simulink model "ClimaEgyMgr"  (R2022b)
settings: Solver=FixedStepDiscrete  StartTime=0.0  StopTime=10.0  FixedStep=0.2  RelTol=1e-3
(part 1 of 2)
settings Model: Creator=blue.ge  LastModifiedBy=blue.ge
settings DataIOCC: SaveFormat=StructureWithTime
settings OptimizationCC: DefaultParameterBehavior=Inlined  EnhancedBackFolding=on  PassReuseOutputArgsAs=Individual arguments  ZeroExternalMemoryAtStartup=off  ZeroInternalMemoryAtStartup=off  OptimizeBlockOrder=speed  DifferentSizesBufferReuse=on  OptimizationCustomize=off  EfficientTunableParamExpr=on
settings DebuggingCC: ParameterTunabilityLossMsg=error
settings ModelReferenceCC: ModelReferenceNumInstancesAllowed=Single
settings SFSimCC: MATLABDynamicMemAlloc=off
settings RTWCC: Description=AUTOSAR  SystemTargetFile=autosar.tlc  GenCodeOnly=on  TemplateMakefile=ert_default_tmf
settings CodeAppCC: ForceParamTrailComments=on  IgnoreCustomStorageClasses=off  OperatorAnnotations=on  SimulinkDataObjDesc=on  SFDataObjDesc=on  InsertBlockDesc=on
settings STFCustomTargetCC: TargetLangStandard=C99 (ISO)  MultiwordLength=256  ParMdlRefBuildCompliant=on  CompOptLevelCompliant=on  IncludeMdlTerminateFcn=off  CombineOutputUpdateFcns=on  SuppressErrorStatus=on  ERTCustomFileBanners=on  SupportNonFinite=off  SupportComplex=off  SupportContinuousTime=off  SupportNonInlinedSFcns=off  RemoveResetFunc=on  MATLABClassNameForMDSCustomization=Simulink.SoftwareTarget.ERTCustomization  AutosarCompliant=on  UseToolchainInfoCompliant=on  LookupTableObjectStructAxisOrder=2,1,3,4,...  LUTObjectStructOrderEvenSpacing=Size,Table,Breakpoints  SystemTargetFile=autosar.tlc
settings ERTTargetCC: TargetLangStandard=C99 (ISO)  ConcurrentExecutionCompliant=off  IncludeMdlTerminateFcn=off  SuppressErrorStatus=on  SupportNonFinite=off  SupportComplex=off  ModelStepFunctionPrototypeControlCompliant=off  CPPClassGenCompliant=off  AutosarCompliant=on  LookupTableObjectStructAxisOrder=2,1,3,4,...  LUTObjectStructOrderEvenSpacing=Size,Table,Breakpoints  InlinedParameterPlacement=NonHierarchical  ERTCustomFileTemplate=example_file_process.tlc

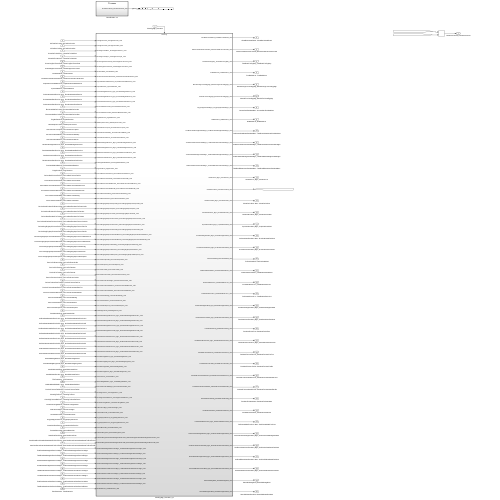
[thumbnail: Simulink's stored preview of the top-level diagram]
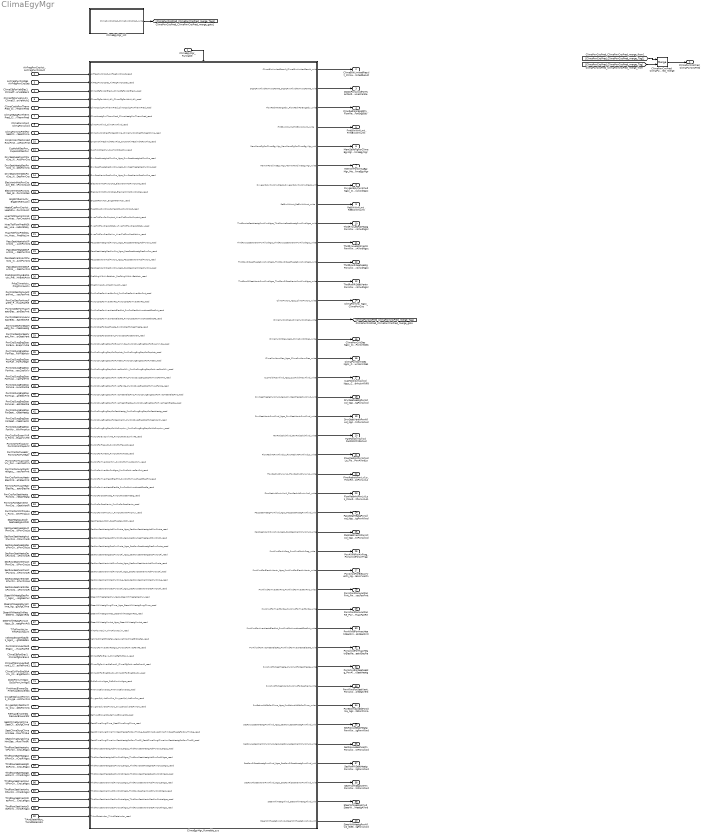
== system ClimaEgyMgr ==
  ClimaEgyMgr_Runnable : Inport  SampleTime=0.2  OutputFunctionCall=on
  AirFragPwrCnsAct_AirFragPwrCnsAct : Inport  Port=2  OutDataTypeStr=Pwr33  PortDimensions=1
  AirFragPwrCnsDes_AirFragPwrCnsDes : Inport  Port=3  OutDataTypeStr=Pwr33  PortDimensions=1
  ClimaCfgForVehElec1_ClimaCfgForVehElec1 : Inport  Port=4  OutDataTypeStr=Boolean  PortDimensions=1
  ClimaCfgForVehHyb1_ClimaCfgForVehHyb1 : Inport  Port=5  OutDataTypeStr=Boolean  PortDimensions=1
  ClimaCoolgPwrThermPred_ClimaCoolgPwrThermPred : Inport  Port=6  OutDataTypeStr=Bus: ClimaCoolgPwrThermPred  PortDimensions=1  BusOutputAsStruct=on
  ClimaHeatgPwrThermPred_ClimaHeatgPwrThermPred : Inport  Port=7  OutDataTypeStr=Bus: ClimaHeatgPwrThermPred  PortDimensions=1  BusOutputAsStruct=on
  ClimaPwrAllwd_ClimaPwrAllwd : Inport  Port=8  OutMin=0  OutMax=2047  OutDataTypeStr=Pwr30  PortDimensions=1
  ClimaPwrCnsPredForSeatClima_ClimaPwrCnsPredForSeatClima : Inport  Port=9  OutDataTypeStr=Bus: ClimaPwrCnsPredRec1  PortDimensions=1  BusOutputAsStruct=on
  CmptmtAirTEstimdAtRowFirst_CmptmtAirTEstimdAtRowFirst : Inport  Port=10  OutDataTypeStr=T30  PortDimensions=1
  CupHoldrDesPwr_CupHoldrDesPwr : Inport  Port=11  OutDataTypeStr=UInt7  PortDimensions=1
  DrvrSeatHeatgActlPwrCns_Ngcc_DrvrSeatHeatgActlPwrCns : Inport  Port=12  OutDataTypeStr=UInt8  PortDimensions=1
  DrvrSeatHeatgDesPwrCns_Ngcc_DrvrSeatHeatgDesPwrCns : Inport  Port=13  OutDataTypeStr=UInt8  PortDimensions=1
  DrvrSeatVentnDesPwrCns_Ngcc_DrvrSeatVentnDesPwrCns : Inport  Port=14  OutDataTypeStr=UInt8  PortDimensions=1
  ElecVentnMotPwrCnsAct_ElecVentnMotPwrCnsAct : Inport  Port=15  OutDataTypeStr=ScrHeatrEgyPwrCns1  PortDimensions=1
  ElecVentnMotPwrCnsDes_ElecVentnMotPwrCnsDes : Inport  Port=16  OutDataTypeStr=ScrHeatrEgyPwrCns1  PortDimensions=1
  EngStrtRemActv_EngStrtRemActv : Inport  Port=17  OutDataTypeStr=Boolean  PortDimensions=1
  HeatdCupPwrCnsAct_HeatdCupPwrCnsAct : Inport  Port=18  OutDataTypeStr=UInt7  PortDimensions=1
  HvacTotFlowForCmptmt_HvacTotFlowForCmptmt : Inport  Port=19  OutDataTypeStr=AirFlow30  PortDimensions=1
  HvacTotFlowPredAtStaby_HvacTotFlowPredAtStaby : Inport  Port=20  OutDataTypeStr=AirFlow30  PortDimensions=1
  HvacTotFlowPredNoLim_HvacTotFlowPredNoLim : Inport  Port=21  OutDataTypeStr=AirFlow30  PortDimensions=1
  PassSeatHeatgActlPwrCns_Ngcc_PassSeatHeatgActlPwrCns : Inport  Port=22  OutDataTypeStr=UInt8  PortDimensions=1
  PassSeatHeatgDesPwrCns_Ngcc_PassSeatHeatgDesPwrCns : Inport  Port=23  OutDataTypeStr=UInt8  PortDimensions=1
  PassSeatVentnActlPwrCns_Ngcc_PassSeatVentnActlPwrCns : Inport  Port=24  OutDataTypeStr=UInt8  PortDimensions=1
  PassSeatVentnDesPwrCns_Ngcc_PassSeatVentnDesPwrCns : Inport  Port=25  OutDataTypeStr=UInt8  PortDimensions=1
  PreCdngWithHvBattActv_PreCdngWithHvBattActv : Inport  Port=26  OutDataTypeStr=Boolean  PortDimensions=1
  PrkgClimaActv_PrkgClimaActv : Inport  Port=27  OutDataTypeStr=Enum: PrkgClimaActv  PortDimensions=1
  PwrCnsDesForHvacFanFrnt_PwrCnsDesForHvacFanFrnt : Inport  Port=28  OutDataTypeStr=Pwr33  PortDimensions=1
  PwrCnsDesForHvacFanRe_PwrCnsDesForHvacFanRe : Inport  Port=29  OutDataTypeStr=Pwr33  PortDimensions=1
  PwrCnsDesForHvacHeatrElecFrnt_PwrCnsDesForHvacHeatrElecFrnt : Inport  Port=30  OutDataTypeStr=Pwr33  PortDimensions=1
  PwrCnsDesForHvacHeatrElecRe_PwrCnsDesForHvacHeatrElecRe : Inport  Port=31  OutDataTypeStr=Pwr33  PortDimensions=1
  PwrCnsDesForSeatHeatg_PwrCnsDesForSeatHeatg : Inport  Port=32  OutDataTypeStr=Pwr33  PortDimensions=1
  PwrCnsDesForSeatVent_PwrCnsDesForSeatVent : Inport  Port=33  OutDataTypeStr=Pwr33  PortDimensions=1
  PwrCnsDurgEngStopForEvaprVlvRe_PwrCnsDurgEngStopForEvaprVlvRe : Inport  Port=34  OutDataTypeStr=Pwr33  PortDimensions=1
  PwrCnsDurgEngStopForFlapActr_PwrCnsDurgEngStopForFlapActr : Inport  Port=35  OutDataTypeStr=Pwr33  PortDimensions=1
  PwrCnsDurgEngStopForFuHeatr_PwrCnsDurgEngStopForFuHeatr : Inport  Port=36  OutDataTypeStr=Pwr33  PortDimensions=1
  PwrCnsDurgEngStopForHvacCooltVlv_PwrCnsDurgEngStopForHvacCooltVlv : Inport  Port=37  OutDataTypeStr=Pwr33  PortDimensions=1
  PwrCnsDurgEngStopForHvacFanFrnt_PwrCnsDurgEngStopForHvacFanFrnt : Inport  Port=38  OutDataTypeStr=Pwr33  PortDimensions=1
  PwrCnsDurgEngStopForHvacFanRe_PwrCnsDurgEngStopForHvacFanRe : Inport  Port=39  OutDataTypeStr=Pwr33  PortDimensions=1
  PwrCnsDurgEngStopForHvacHeatrElecFrnt_PwrCnsDurgEngStopForHvacHeatrElecFrnt : Inport  Port=40  OutDataTypeStr=Pwr33  PortDimensions=1
  PwrCnsDurgEngStopForHvacHeatrElecRe_PwrCnsDurgEngStopForHvacHeatrElecRe : Inport  Port=41  OutDataTypeStr=Pwr33  PortDimensions=1
  PwrCnsDurgEngStopForSeatHeatg_PwrCnsDurgEngStopForSeatHeatg : Inport  Port=42  OutDataTypeStr=Pwr33  PortDimensions=1
  PwrCnsDurgEngStopForSeatVentn_PwrCnsDurgEngStopForSeatVentn : Inport  Port=43  OutDataTypeStr=Pwr33  PortDimensions=1
  PwrCnsDurgEngStopForWtrPmpAux_PwrCnsDurgEngStopForWtrPmpAux : Inport  Port=44  OutDataTypeStr=Pwr33  PortDimensions=1
  PwrCnsForEvaprVlvRe_PwrCnsForEvaprVlvRe : Inport  Port=45  OutDataTypeStr=Pwr33  PortDimensions=1
  PwrCnsForFlapActr_PwrCnsForFlapActr : Inport  Port=46  OutDataTypeStr=Pwr33  PortDimensions=1
  PwrCnsForFuHeatr_PwrCnsForFuHeatr : Inport  Port=47  OutDataTypeStr=Pwr33  PortDimensions=1
  PwrCnsForHvacCooltVlv_PwrCnsForHvacCooltVlv : Inport  Port=48  OutDataTypeStr=Pwr33  PortDimensions=1
  PwrCnsForHvacFanFrntNgcc_PwrCnsForHvacFanFrnt : Inport  Port=49  OutDataTypeStr=Pwr33  PortDimensions=1
  PwrCnsForHvacHeatrElecFrnt_PwrCnsForHvacHeatrElecFrnt : Inport  Port=50  OutDataTypeStr=Pwr33  PortDimensions=1
  PwrCnsForHvacHeatrElecRe_PwrCnsForHvacHeatrElecRe : Inport  Port=51  OutDataTypeStr=Pwr33  PortDimensions=1
  PwrCnsForSeatHeatg_PwrCnsForSeatHeatg : Inport  Port=52  OutDataTypeStr=Pwr33  PortDimensions=1
  PwrCnsForSeatVentn_PwrCnsForSeatVentn : Inport  Port=53  OutDataTypeStr=Pwr33  PortDimensions=1
  PwrCnsForWtrPmpAux_PwrCnsForWtrPmpAux : Inport  Port=54  OutDataTypeStr=Pwr33  PortDimensions=1
  SeatHeatgAutCdn_SeatHeatgAutCdn : Inport  Port=55  OutDataTypeStr=Enum: Flg1  PortDimensions=1
  SecRowSeatHeatgActlPwrCnsLe_Ngcc_SecRowSeatHeatgActlPwrCnsLe : Inport  Port=56  OutDataTypeStr=UInt8  PortDimensions=1
  SecRowSeatHeatgActlPwrCnsRi_Ngcc_SecRowSeatHeatgActlPwrCnsRi : Inport  Port=57  OutDataTypeStr=UInt8  PortDimensions=1
  SecRowSeatHeatgDesPwrCnsLe_Ngcc_SecRowSeatHeatgDesPwrCnsLe : Inport  Port=58  OutDataTypeStr=UInt8  PortDimensions=1
  SecRowSeatHeatgDesPwrCnsRi_Ngcc_SecRowSeatHeatgDesPwrCnsRi : Inport  Port=59  OutDataTypeStr=UInt8  PortDimensions=1
  SecRowSeatVentnActlPwrCnsLe_Ngcc_SecRowSeatVentnActlPwrCnsLe : Inport  Port=60  OutDataTypeStr=UInt8  PortDimensions=1
  SecRowSeatVentnActlPwrCnsRi_Ngcc_SecRowSeatVentnActlPwrCnsRi : Inport  Port=61  OutDataTypeStr=UInt8  PortDimensions=1
  SecRowSeatVentnDesPwrCnsLe_Ngcc_SecRowSeatVentnDesPwrCnsLe : Inport  Port=62  OutDataTypeStr=UInt8  PortDimensions=1
  SecRowSeatVentnDesPwrCnsRi_Ngcc_SecRowSeatVentnDesPwrCnsRi : Inport  Port=63  OutDataTypeStr=UInt8  PortDimensions=1
  SteerWhlHeatgDesPwr_Ngcc_SteerWhlHeatgDesPwr : Inport  Port=64  OutDataTypeStr=UInt8  PortDimensions=1
  SteerWhlHeatgDurgClima_Ngcc_SteerWhlHeatgDurgClima : Inport  Port=65  OutDataTypeStr=Boolean  PortDimensions=1
  SteerWhlHeatgOnReq_SteerWhlHeatgOnReq : Inport  Port=66  OutDataTypeStr=Enum: SteerWhlHeatgOnCmdTyp  PortDimensions=1
  SteerWhlHeatgPwrAct_Ngcc_SteerWhlHeatgPwrAct : Inport  Port=67  OutDataTypeStr=UInt8  PortDimensions=1
  TiToFlowNoLim_TiToFlowNoLim : Inport  Port=68  OutDataTypeStr=Ti33  PortDimensions=1
  VehModMngtGlbSafe1_Ngcc_VehModMngtGlbSafe1 : Inport  Port=69  OutDataTypeStr=Bus: VehModMngtGlbSafe1  PortDimensions=1  BusOutputAsStruct=on
  PwrCnsForHvacFanReNgcc_PwrCnsForHvacFanRe : Inport  Port=70  OutDataTypeStr=Pwr33  PortDimensions=1
  ClimaCfgForErev1_ClimaCfgForErev1 : Inport  Port=71  OutDataTypeStr=Boolean  PortDimensions=1
  ClimaCfgForHvacRePrsnt1_ClimaCfgForHvacRePrsnt1 : Inport  Port=72  OutDataTypeStr=Enum: CarCfgForHvacRe  PortDimensions=1
  ClimaCtrlForEngStbActv_ClimaCtrlForEngStbActv : Inport  Port=73  OutDataTypeStr=Boolean  PortDimensions=1
  DcDcPwrLimNgcc_DcDcPwrLimNgcc : Inport  Port=74  OutDataTypeStr=Boolean  PortDimensions=1
  FrntHvacBlowerSts_FrntHvacBlowerSts : Inport  Port=75  OutDataTypeStr=Enum: HvacFanSts  PortDimensions=1
  OxygenSplyActPwrCns_OxygenSplyActPwrCns : Inport  Port=76  OutMin=0  OutMax=2047  OutDataTypeStr=Pwr30  PortDimensions=1
  OxygenSplyDesPwrCns_OxygenSplyDesPwrCns : Inport  Port=77  OutMin=0  OutMax=2047  OutDataTypeStr=Pwr30  PortDimensions=1
  ReHvacBlowerSts_ReHvacBlowerSts : Inport  Port=78  OutDataTypeStr=Enum: HvacFanSts  PortDimensions=1
  SeatClimaDurgClima_SeatClimaDurgClima : Inport  Port=79  OutDataTypeStr=Bus: HmiSeatClima  PortDimensions=1  BusOutputAsStruct=on
  SeatClimaDurgClimaHmiSeatHeatgForRowThrdLe_SeatClimaDurgClimaHmiSeatHeatgForRowThrdLe : Inport  Port=80  OutDataTypeStr=Enum: SeatClimaLvl  PortDimensions=1
  SeatClimaDurgClimaHmiSeatHeatgForRowThrdRi_SeatClimaDurgClimaHmiSeatHeatgForRowThrdRi : Inport  Port=81  OutDataTypeStr=Enum: SeatClimaLvl  PortDimensions=1
  ThrdRowSeatHeatgActlPwrCnsLeNgcc_ThrdRowSeatHeatgActlPwrCnsLeNgcc : Inport  Port=82  OutDataTypeStr=UInt8  PortDimensions=1
  ThrdRowSeatHeatgActlPwrCnsRiNgcc_ThrdRowSeatHeatgActlPwrCnsRiNgcc : Inport  Port=83  OutDataTypeStr=UInt8  PortDimensions=1
  ThrdRowSeatHeatgDesPwrCnsLeNgcc_ThrdRowSeatHeatgDesPwrCnsLeNgcc : Inport  Port=84  OutDataTypeStr=UInt8  PortDimensions=1
  ThrdRowSeatHeatgDesPwrCnsRiNgcc_ThrdRowSeatHeatgDesPwrCnsRiNgcc : Inport  Port=85  OutDataTypeStr=UInt8  PortDimensions=1
  ThrdRowSeatVentnActlPwrCnsLeNgcc_ThrdRowSeatVentnActlPwrCnsLeNgcc : Inport  Port=86  OutDataTypeStr=UInt8  PortDimensions=1
  ThrdRowSeatVentnActlPwrCnsRiNgcc_ThrdRowSeatVentnActlPwrCnsRiNgcc : Inport  Port=87  OutDataTypeStr=UInt8  PortDimensions=1
  ThrdRowSeatVentnDesPwrCnsLeNgcc_ThrdRowSeatVentnDesPwrCnsLeNgcc : Inport  Port=88  OutDataTypeStr=UInt8  PortDimensions=1
  ThrdRowSeatVentnDesPwrCnsRiNgcc_ThrdRowSeatVentnDesPwrCnsRiNgcc : Inport  Port=89  OutDataTypeStr=UInt8  PortDimensions=1
  TiAndDateIndcn_TiAndDateIndcn : Inport  Port=90  OutDataTypeStr=Bus: DateTi30  PortDimensions=1  BusOutputAsStruct=on
  ClimaEgyMgr_Init : Subsystem
  ClimaEgyMgr_Runnable_sys : Subsystem
  ClimaPwrCnsPred_ClimaPwrCnsPred_merge : Merge
  ClimaPwrCnsPred_ClimaPwrCnsPred : Outport  OutDataTypeStr=Bus: ClimaPwrCnsPredRec1  PortDimensions=1  BusOutputAsStruct=on
  ClimaBlwLimitedResult_ClimaBlwLimitedResult : Outport  Port=2  OutDataTypeStr=Boolean  PortDimensions=1
  DCDCPwrAllwdForHvacFanRe_DCDCPwrAllwdForHvacFanRe : Outport  Port=3  OutDataTypeStr=UInt8  PortDimensions=1
  FlowRednForEngStby_FlowRednForEngStby : Outport  Port=4  OutDataTypeStr=Boolean  PortDimensions=1
  FrntBlwWrkLmt_FrntBlwWrkLmt : Outport  Port=5  OutDataTypeStr=UInt8  PortDimensions=1
  MemSaveFlgForClimaEgyMgr_MemSaveFlgForClimaEgyMgr : Outport  Port=6  OutDataTypeStr=Boolean  PortDimensions=1
  MemWrForClimaEgyMgr_MemWrForClimaEgyMgr : Outport  Port=7  OutDataTypeStr=Bus: MemRecForClimaEgyMgr  PortDimensions=1  BusOutputAsStruct=on
  OxygenSplyPwrAllwdNgcc_OxygenSplyPwrAllwdNgcc : Outport  Port=8  OutMin=0  OutMax=2047  OutDataTypeStr=Pwr30  PortDimensions=1
  ReBlwWrkLmt_ReBlwWrkLmt : Outport  Port=9  OutDataTypeStr=UInt8  PortDimensions=1
  ThrdRowLeSeatHeatgPwrAllwdNgcc_ThrdRowLeSeatHeatgPwrAllwdNgcc : Outport  Port=10  OutDataTypeStr=UInt8  PortDimensions=1
  ThrdRowLeSeatVentnPwrAllwdNgcc_ThrdRowLeSeatVentnPwrAllwdNgcc : Outport  Port=11  OutDataTypeStr=UInt8  PortDimensions=1
  ThrdRowRiSeatHeatgPwrAllwdNgcc_ThrdRowRiSeatHeatgPwrAllwdNgcc : Outport  Port=12  OutDataTypeStr=UInt8  PortDimensions=1
  ThrdRowRiSeatVentnPwrAllwdNgcc_ThrdRowRiSeatVentnPwrAllwdNgcc : Outport  Port=13  OutDataTypeStr=UInt8  PortDimensions=1
  ClimaPwrCns_Ngcc_ClimaPwrCns : Outport  Port=14  OutMin=0  OutMax=2047  OutDataTypeStr=Pwr30  PortDimensions=1
  ClimaPwrCritDes_Ngcc_ClimaPwrCritDes : Outport  Port=15  OutMin=0  OutMax=2047  OutDataTypeStr=Pwr30  PortDimensions=1
  ClimaPwrNormDes_Ngcc_ClimaPwrNormDes : Outport  Port=16  OutMin=0  OutMax=2047  OutDataTypeStr=Pwr30  PortDimensions=1
  CupHoldrActvAllwd_Ngcc_CupHoldrActvAllwd : Outport  Port=17  OutDataTypeStr=ScrHeatrEgyPwrCns1  PortDimensions=1
  DrvrSeatHeatgPwrAllwd_Ngcc_DrvrSeatHeatgPwrAllwd : Outport  Port=18  OutDataTypeStr=UInt8  PortDimensions=1
  DrvrSeatVentnPwrAllwd_Ngcc_DrvrSeatVentnPwrAllwd : Outport  Port=19  OutDataTypeStr=UInt8  PortDimensions=1
  FanPotiCtrldAllwd_FanPotiCtrldAllwd : Outport  Port=20  OutDataTypeStr=Boolean  PortDimensions=1
  FlowRednAtPwrAllwdLo_FlowRednAtPwrAllwdLo : Outport  Port=21  OutDataTypeStr=Boolean  PortDimensions=1
  FlowRednAtPwrLvlLo_FlowRednAtPwrLvlLo : Outport  Port=22  OutDataTypeStr=Boolean  PortDimensions=1
  FlowRednAtPwrLvlLo1_FlowRednAtPwrLvlLo1 : Outport  Port=23  OutDataTypeStr=Boolean  PortDimensions=1
  PassSeatHeatgPwrAllwd_Ngcc_PassSeatHeatgPwrAllwd : Outport  Port=24  OutDataTypeStr=UInt8  PortDimensions=1
  PassSeatVentnPwrAllwd_Ngcc_PassSeatVentnPwrAllwd : Outport  Port=25  OutDataTypeStr=UInt8  PortDimensions=1
  PwrAllwdForAirFrag_PwrAllwdForAirFrag : Outport  Port=26  OutDataTypeStr=Pwr33  PortDimensions=1
  PwrAllwdForElecAirVentn_Ngcc_PwrAllwdForElecAirVentn : Outport  Port=27  OutDataTypeStr=ScrHeatrEgyPwrCns1  PortDimensions=1
  PwrAllwdForHvacFanFrnt_PwrAllwdForHvacFanFrnt : Outport  Port=28  OutDataTypeStr=Pwr33  PortDimensions=1
  PwrAllwdForHvacFanRe_PwrAllwdForHvacFanRe : Outport  Port=29  OutDataTypeStr=Pwr33  PortDimensions=1
  PwrAllwdForHvacHeatrElecFrnt_PwrAllwdForHvacHeatrElecFrnt : Outport  Port=30  OutDataTypeStr=Pwr33  PortDimensions=1
  PwrAllwdForHvacHeatrElecRe_PwrAllwdForHvacHeatrElecRe : Outport  Port=31  OutDataTypeStr=Pwr33  PortDimensions=1
  PwrAllwdForSeatHeatg_PwrAllwdForSeatHeatg : Outport  Port=32  OutDataTypeStr=Pwr33  PortDimensions=1
  PwrAllwdForSeatVent_PwrAllwdForSeatVent : Outport  Port=33  OutDataTypeStr=Pwr33  PortDimensions=1
  PwrEstimdAtStbForClima_Ngcc_PwrEstimdAtStbForClima : Outport  Port=34  OutMin=0  OutMax=2047  OutDataTypeStr=Pwr30  PortDimensions=1
  SecRowLeSeatHeatgPwrAllwd_Ngcc_SecRowLeSeatHeatgPwrAllwd : Outport  Port=35  OutDataTypeStr=UInt8  PortDimensions=1
  SecRowLeSeatVentnPwrAllwd_Ngcc_SecRowLeSeatVentnPwrAllwd : Outport  Port=36  OutDataTypeStr=UInt8  PortDimensions=1
  SecRowRiSeatHeatgPwrAllwd_Ngcc_SecRowRiSeatHeatgPwrAllwd : Outport  Port=37  OutDataTypeStr=UInt8  PortDimensions=1
  SecRowRiSeatVentnPwrAllwd_Ngcc_SecRowRiSeatVentnPwrAllwd : Outport  Port=38  OutDataTypeStr=UInt8  PortDimensions=1
  SteerWhlHeatgAllwd_SteerWhlHeatgAllwd : Outport  Port=39  OutDataTypeStr=ClimaLoadPwr  PortDimensions=1
  SteerWhlHeatgPwrAllwd_SteerWhlHeatgPwrAllwd : Outport  Port=40  OutDataTypeStr=UInt8  PortDimensions=1
  wires:
  AirFragPwrCnsAct_AirFragPwrCnsAct/1 -> ClimaEgyMgr_Runnable_sys/1(AirFragPwrCnsAct_AirFragPwrCnsAct_read)
  AirFragPwrCnsDes_AirFragPwrCnsDes/1 -> ClimaEgyMgr_Runnable_sys/2(AirFragPwrCnsDes_AirFragPwrCnsDes_read)
  ClimaCfgForVehElec1_ClimaCfgForVehElec1/1 -> ClimaEgyMgr_Runnable_sys/3(ClimaCfgForVehElec1_ClimaCfgForVehElec1_read)
  ClimaCfgForVehHyb1_ClimaCfgForVehHyb1/1 -> ClimaEgyMgr_Runnable_sys/4(ClimaCfgForVehHyb1_ClimaCfgForVehHyb1_read)
  ClimaCoolgPwrThermPred_ClimaCoolgPwrThermPred/1 -> ClimaEgyMgr_Runnable_sys/5(ClimaCoolgPwrThermPred_ClimaCoolgPwrThermPred_read)
  ClimaHeatgPwrThermPred_ClimaHeatgPwrThermPred/1 -> ClimaEgyMgr_Runnable_sys/6(ClimaHeatgPwrThermPred_ClimaHeatgPwrThermPred_read)
  ClimaPwrAllwd_ClimaPwrAllwd/1 -> ClimaEgyMgr_Runnable_sys/7(ClimaPwrAllwd_ClimaPwrAllwd_read)
  ClimaPwrCnsPredForSeatClima_ClimaPwrCnsPredForSeatClima/1 -> ClimaEgyMgr_Runnable_sys/8(ClimaPwrCnsPredForSeatClima_ClimaPwrCnsPredForSeatClima_read)
  CmptmtAirTEstimdAtRowFirst_CmptmtAirTEstimdAtRowFirst/1 -> ClimaEgyMgr_Runnable_sys/9(CmptmtAirTEstimdAtRowFirst_CmptmtAirTEstimdAtRowFirst_read)
  CupHoldrDesPwr_CupHoldrDesPwr/1 -> ClimaEgyMgr_Runnable_sys/10(CupHoldrDesPwr_CupHoldrDesPwr_read)
  DrvrSeatHeatgActlPwrCns_Ngcc_DrvrSeatHeatgActlPwrCns/1 -> ClimaEgyMgr_Runnable_sys/11(DrvrSeatHeatgActlPwrCns_Ngcc_DrvrSeatHeatgActlPwrCns_read)
  DrvrSeatHeatgDesPwrCns_Ngcc_DrvrSeatHeatgDesPwrCns/1 -> ClimaEgyMgr_Runnable_sys/12(DrvrSeatHeatgDesPwrCns_Ngcc_DrvrSeatHeatgDesPwrCns_read)
  DrvrSeatVentnDesPwrCns_Ngcc_DrvrSeatVentnDesPwrCns/1 -> ClimaEgyMgr_Runnable_sys/13(DrvrSeatVentnDesPwrCns_Ngcc_DrvrSeatVentnDesPwrCns_read)
  ElecVentnMotPwrCnsAct_ElecVentnMotPwrCnsAct/1 -> ClimaEgyMgr_Runnable_sys/14(ElecVentnMotPwrCnsAct_ElecVentnMotPwrCnsAct_read)
  ElecVentnMotPwrCnsDes_ElecVentnMotPwrCnsDes/1 -> ClimaEgyMgr_Runnable_sys/15(ElecVentnMotPwrCnsDes_ElecVentnMotPwrCnsDes_read)
  EngStrtRemActv_EngStrtRemActv/1 -> ClimaEgyMgr_Runnable_sys/16(EngStrtRemActv_EngStrtRemActv_read)
  HeatdCupPwrCnsAct_HeatdCupPwrCnsAct/1 -> ClimaEgyMgr_Runnable_sys/17(HeatdCupPwrCnsAct_HeatdCupPwrCnsAct_read)
  HvacTotFlowForCmptmt_HvacTotFlowForCmptmt/1 -> ClimaEgyMgr_Runnable_sys/18(HvacTotFlowForCmptmt_HvacTotFlowForCmptmt_read)
  HvacTotFlowPredAtStaby_HvacTotFlowPredAtStaby/1 -> ClimaEgyMgr_Runnable_sys/19(HvacTotFlowPredAtStaby_HvacTotFlowPredAtStaby_read)
  HvacTotFlowPredNoLim_HvacTotFlowPredNoLim/1 -> ClimaEgyMgr_Runnable_sys/20(HvacTotFlowPredNoLim_HvacTotFlowPredNoLim_read)
  PassSeatHeatgActlPwrCns_Ngcc_PassSeatHeatgActlPwrCns/1 -> ClimaEgyMgr_Runnable_sys/21(PassSeatHeatgActlPwrCns_Ngcc_PassSeatHeatgActlPwrCns_read)
  PassSeatHeatgDesPwrCns_Ngcc_PassSeatHeatgDesPwrCns/1 -> ClimaEgyMgr_Runnable_sys/22(PassSeatHeatgDesPwrCns_Ngcc_PassSeatHeatgDesPwrCns_read)
  PassSeatVentnActlPwrCns_Ngcc_PassSeatVentnActlPwrCns/1 -> ClimaEgyMgr_Runnable_sys/23(PassSeatVentnActlPwrCns_Ngcc_PassSeatVentnActlPwrCns_read)
  PassSeatVentnDesPwrCns_Ngcc_PassSeatVentnDesPwrCns/1 -> ClimaEgyMgr_Runnable_sys/24(PassSeatVentnDesPwrCns_Ngcc_PassSeatVentnDesPwrCns_read)
  PreCdngWithHvBattActv_PreCdngWithHvBattActv/1 -> ClimaEgyMgr_Runnable_sys/25(PreCdngWithHvBattActv_PreCdngWithHvBattActv_read)
  PrkgClimaActv_PrkgClimaActv/1 -> ClimaEgyMgr_Runnable_sys/26(PrkgClimaActv_PrkgClimaActv_read)
  PwrCnsDesForHvacFanFrnt_PwrCnsDesForHvacFanFrnt/1 -> ClimaEgyMgr_Runnable_sys/27(PwrCnsDesForHvacFanFrnt_PwrCnsDesForHvacFanFrnt_read)
  PwrCnsDesForHvacFanRe_PwrCnsDesForHvacFanRe/1 -> ClimaEgyMgr_Runnable_sys/28(PwrCnsDesForHvacFanRe_PwrCnsDesForHvacFanRe_read)
  PwrCnsDesForHvacHeatrElecFrnt_PwrCnsDesForHvacHeatrElecFrnt/1 -> ClimaEgyMgr_Runnable_sys/29(PwrCnsDesForHvacHeatrElecFrnt_PwrCnsDesForHvacHeatrElecFrnt_read)
  PwrCnsDesForHvacHeatrElecRe_PwrCnsDesForHvacHeatrElecRe/1 -> ClimaEgyMgr_Runnable_sys/30(PwrCnsDesForHvacHeatrElecRe_PwrCnsDesForHvacHeatrElecRe_read)
  PwrCnsDesForSeatHeatg_PwrCnsDesForSeatHeatg/1 -> ClimaEgyMgr_Runnable_sys/31(PwrCnsDesForSeatHeatg_PwrCnsDesForSeatHeatg_read)
  PwrCnsDesForSeatVent_PwrCnsDesForSeatVent/1 -> ClimaEgyMgr_Runnable_sys/32(PwrCnsDesForSeatVent_PwrCnsDesForSeatVent_read)
  PwrCnsDurgEngStopForEvaprVlvRe_PwrCnsDurgEngStopForEvaprVlvRe/1 -> ClimaEgyMgr_Runnable_sys/33(PwrCnsDurgEngStopForEvaprVlvRe_PwrCnsDurgEngStopForEvaprVlvRe_read)
  PwrCnsDurgEngStopForFlapActr_PwrCnsDurgEngStopForFlapActr/1 -> ClimaEgyMgr_Runnable_sys/34(PwrCnsDurgEngStopForFlapActr_PwrCnsDurgEngStopForFlapActr_read)
  PwrCnsDurgEngStopForFuHeatr_PwrCnsDurgEngStopForFuHeatr/1 -> ClimaEgyMgr_Runnable_sys/35(PwrCnsDurgEngStopForFuHeatr_PwrCnsDurgEngStopForFuHeatr_read)
  PwrCnsDurgEngStopForHvacCooltVlv_PwrCnsDurgEngStopForHvacCooltVlv/1 -> ClimaEgyMgr_Runnable_sys/36(PwrCnsDurgEngStopForHvacCooltVlv_PwrCnsDurgEngStopForHvacCooltVlv_read)
  PwrCnsDurgEngStopForHvacFanFrnt_PwrCnsDurgEngStopForHvacFanFrnt/1 -> ClimaEgyMgr_Runnable_sys/37(PwrCnsDurgEngStopForHvacFanFrnt_PwrCnsDurgEngStopForHvacFanFrnt_read)
  PwrCnsDurgEngStopForHvacFanRe_PwrCnsDurgEngStopForHvacFanRe/1 -> ClimaEgyMgr_Runnable_sys/38(PwrCnsDurgEngStopForHvacFanRe_PwrCnsDurgEngStopForHvacFanRe_read)
  PwrCnsDurgEngStopForHvacHeatrElecFrnt_PwrCnsDurgEngStopForHvacHeatrElecFrnt/1 -> ClimaEgyMgr_Runnable_sys/39(PwrCnsDurgEngStopForHvacHeatrElecFrnt_PwrCnsDurgEngStopForHvacHeatrElecFrnt_read)
  PwrCnsDurgEngStopForHvacHeatrElecRe_PwrCnsDurgEngStopForHvacHeatrElecRe/1 -> ClimaEgyMgr_Runnable_sys/40(PwrCnsDurgEngStopForHvacHeatrElecRe_PwrCnsDurgEngStopForHvacHeatrElecRe_read)
  PwrCnsDurgEngStopForSeatHeatg_PwrCnsDurgEngStopForSeatHeatg/1 -> ClimaEgyMgr_Runnable_sys/41(PwrCnsDurgEngStopForSeatHeatg_PwrCnsDurgEngStopForSeatHeatg_read)
  PwrCnsDurgEngStopForSeatVentn_PwrCnsDurgEngStopForSeatVentn/1 -> ClimaEgyMgr_Runnable_sys/42(PwrCnsDurgEngStopForSeatVentn_PwrCnsDurgEngStopForSeatVentn_read)
  PwrCnsDurgEngStopForWtrPmpAux_PwrCnsDurgEngStopForWtrPmpAux/1 -> ClimaEgyMgr_Runnable_sys/43(PwrCnsDurgEngStopForWtrPmpAux_PwrCnsDurgEngStopForWtrPmpAux_read)
  PwrCnsForEvaprVlvRe_PwrCnsForEvaprVlvRe/1 -> ClimaEgyMgr_Runnable_sys/44(PwrCnsForEvaprVlvRe_PwrCnsForEvaprVlvRe_read)
  PwrCnsForFlapActr_PwrCnsForFlapActr/1 -> ClimaEgyMgr_Runnable_sys/45(PwrCnsForFlapActr_PwrCnsForFlapActr_read)
  PwrCnsForFuHeatr_PwrCnsForFuHeatr/1 -> ClimaEgyMgr_Runnable_sys/46(PwrCnsForFuHeatr_PwrCnsForFuHeatr_read)
  PwrCnsForHvacCooltVlv_PwrCnsForHvacCooltVlv/1 -> ClimaEgyMgr_Runnable_sys/47(PwrCnsForHvacCooltVlv_PwrCnsForHvacCooltVlv_read)
  PwrCnsForHvacFanFrntNgcc_PwrCnsForHvacFanFrnt/1 -> ClimaEgyMgr_Runnable_sys/48(PwrCnsForHvacFanFrntNgcc_PwrCnsForHvacFanFrnt_read)
  PwrCnsForHvacHeatrElecFrnt_PwrCnsForHvacHeatrElecFrnt/1 -> ClimaEgyMgr_Runnable_sys/49(PwrCnsForHvacHeatrElecFrnt_PwrCnsForHvacHeatrElecFrnt_read)
  PwrCnsForHvacHeatrElecRe_PwrCnsForHvacHeatrElecRe/1 -> ClimaEgyMgr_Runnable_sys/50(PwrCnsForHvacHeatrElecRe_PwrCnsForHvacHeatrElecRe_read)
  PwrCnsForSeatHeatg_PwrCnsForSeatHeatg/1 -> ClimaEgyMgr_Runnable_sys/51(PwrCnsForSeatHeatg_PwrCnsForSeatHeatg_read)
  PwrCnsForSeatVentn_PwrCnsForSeatVentn/1 -> ClimaEgyMgr_Runnable_sys/52(PwrCnsForSeatVentn_PwrCnsForSeatVentn_read)
  PwrCnsForWtrPmpAux_PwrCnsForWtrPmpAux/1 -> ClimaEgyMgr_Runnable_sys/53(PwrCnsForWtrPmpAux_PwrCnsForWtrPmpAux_read)
  SeatHeatgAutCdn_SeatHeatgAutCdn/1 -> ClimaEgyMgr_Runnable_sys/54(SeatHeatgAutCdn_SeatHeatgAutCdn_read)
  SecRowSeatHeatgActlPwrCnsLe_Ngcc_SecRowSeatHeatgActlPwrCnsLe/1 -> ClimaEgyMgr_Runnable_sys/55(SecRowSeatHeatgActlPwrCnsLe_Ngcc_SecRowSeatHeatgActlPwrCnsLe_read)
  SecRowSeatHeatgActlPwrCnsRi_Ngcc_SecRowSeatHeatgActlPwrCnsRi/1 -> ClimaEgyMgr_Runnable_sys/56(SecRowSeatHeatgActlPwrCnsRi_Ngcc_SecRowSeatHeatgActlPwrCnsRi_read)
  SecRowSeatHeatgDesPwrCnsLe_Ngcc_SecRowSeatHeatgDesPwrCnsLe/1 -> ClimaEgyMgr_Runnable_sys/57(SecRowSeatHeatgDesPwrCnsLe_Ngcc_SecRowSeatHeatgDesPwrCnsLe_read)
  SecRowSeatHeatgDesPwrCnsRi_Ngcc_SecRowSeatHeatgDesPwrCnsRi/1 -> ClimaEgyMgr_Runnable_sys/58(SecRowSeatHeatgDesPwrCnsRi_Ngcc_SecRowSeatHeatgDesPwrCnsRi_read)
  SecRowSeatVentnActlPwrCnsLe_Ngcc_SecRowSeatVentnActlPwrCnsLe/1 -> ClimaEgyMgr_Runnable_sys/59(SecRowSeatVentnActlPwrCnsLe_Ngcc_SecRowSeatVentnActlPwrCnsLe_read)
  SecRowSeatVentnActlPwrCnsRi_Ngcc_SecRowSeatVentnActlPwrCnsRi/1 -> ClimaEgyMgr_Runnable_sys/60(SecRowSeatVentnActlPwrCnsRi_Ngcc_SecRowSeatVentnActlPwrCnsRi_read)
  SecRowSeatVentnDesPwrCnsLe_Ngcc_SecRowSeatVentnDesPwrCnsLe/1 -> ClimaEgyMgr_Runnable_sys/61(SecRowSeatVentnDesPwrCnsLe_Ngcc_SecRowSeatVentnDesPwrCnsLe_read)
  SecRowSeatVentnDesPwrCnsRi_Ngcc_SecRowSeatVentnDesPwrCnsRi/1 -> ClimaEgyMgr_Runnable_sys/62(SecRowSeatVentnDesPwrCnsRi_Ngcc_SecRowSeatVentnDesPwrCnsRi_read)
  SteerWhlHeatgDesPwr_Ngcc_SteerWhlHeatgDesPwr/1 -> ClimaEgyMgr_Runnable_sys/63(SteerWhlHeatgDesPwr_Ngcc_SteerWhlHeatgDesPwr_read)
  SteerWhlHeatgDurgClima_Ngcc_SteerWhlHeatgDurgClima/1 -> ClimaEgyMgr_Runnable_sys/64(SteerWhlHeatgDurgClima_Ngcc_SteerWhlHeatgDurgClima_read)
  SteerWhlHeatgOnReq_SteerWhlHeatgOnReq/1 -> ClimaEgyMgr_Runnable_sys/65(SteerWhlHeatgOnReq_SteerWhlHeatgOnReq_read)
  SteerWhlHeatgPwrAct_Ngcc_SteerWhlHeatgPwrAct/1 -> ClimaEgyMgr_Runnable_sys/66(SteerWhlHeatgPwrAct_Ngcc_SteerWhlHeatgPwrAct_read)
  TiToFlowNoLim_TiToFlowNoLim/1 -> ClimaEgyMgr_Runnable_sys/67(TiToFlowNoLim_TiToFlowNoLim_read)
  VehModMngtGlbSafe1_Ngcc_VehModMngtGlbSafe1/1 -> ClimaEgyMgr_Runnable_sys/68(VehModMngtGlbSafe1_Ngcc_VehModMngtGlbSafe1_read)
  PwrCnsForHvacFanReNgcc_PwrCnsForHvacFanRe/1 -> ClimaEgyMgr_Runnable_sys/69(PwrCnsForHvacFanReNgcc_PwrCnsForHvacFanRe_read)
  ClimaCfgForErev1_ClimaCfgForErev1/1 -> ClimaEgyMgr_Runnable_sys/70(ClimaCfgForErev1_ClimaCfgForErev1_read)
  ClimaCfgForHvacRePrsnt1_ClimaCfgForHvacRePrsnt1/1 -> ClimaEgyMgr_Runnable_sys/71(ClimaCfgForHvacRePrsnt1_ClimaCfgForHvacRePrsnt1_read)
  ClimaCtrlForEngStbActv_ClimaCtrlForEngStbActv/1 -> ClimaEgyMgr_Runnable_sys/72(ClimaCtrlForEngStbActv_ClimaCtrlForEngStbActv_read)
  DcDcPwrLimNgcc_DcDcPwrLimNgcc/1 -> ClimaEgyMgr_Runnable_sys/73(DcDcPwrLimNgcc_DcDcPwrLimNgcc_read)
  FrntHvacBlowerSts_FrntHvacBlowerSts/1 -> ClimaEgyMgr_Runnable_sys/74(FrntHvacBlowerSts_FrntHvacBlowerSts_read)
  OxygenSplyActPwrCns_OxygenSplyActPwrCns/1 -> ClimaEgyMgr_Runnable_sys/75(OxygenSplyActPwrCns_OxygenSplyActPwrCns_read)
  OxygenSplyDesPwrCns_OxygenSplyDesPwrCns/1 -> ClimaEgyMgr_Runnable_sys/76(OxygenSplyDesPwrCns_OxygenSplyDesPwrCns_read)
  ReHvacBlowerSts_ReHvacBlowerSts/1 -> ClimaEgyMgr_Runnable_sys/77(ReHvacBlowerSts_ReHvacBlowerSts_read)
  SeatClimaDurgClima_SeatClimaDurgClima/1 -> ClimaEgyMgr_Runnable_sys/78(SeatClimaDurgClima_SeatClimaDurgClima_read)
  SeatClimaDurgClimaHmiSeatHeatgForRowThrdLe_SeatClimaDurgClimaHmiSeatHeatgForRowThrdLe/1 -> ClimaEgyMgr_Runnable_sys/79(SeatClimaDurgClimaHmiSeatHeatgForRowThrdLe_SeatClimaDurgClimaHmiSeatHeatgForRowThrdLe_read)
  SeatClimaDurgClimaHmiSeatHeatgForRowThrdRi_SeatClimaDurgClimaHmiSeatHeatgForRowThrdRi/1 -> ClimaEgyMgr_Runnable_sys/80(SeatClimaDurgClimaHmiSeatHeatgForRowThrdRi_SeatClimaDurgClimaHmiSeatHeatgForRowThrdRi_read)
  ThrdRowSeatHeatgActlPwrCnsLeNgcc_ThrdRowSeatHeatgActlPwrCnsLeNgcc/1 -> ClimaEgyMgr_Runnable_sys/81(ThrdRowSeatHeatgActlPwrCnsLeNgcc_ThrdRowSeatHeatgActlPwrCnsLeNgcc_read)
  ThrdRowSeatHeatgActlPwrCnsRiNgcc_ThrdRowSeatHeatgActlPwrCnsRiNgcc/1 -> ClimaEgyMgr_Runnable_sys/82(ThrdRowSeatHeatgActlPwrCnsRiNgcc_ThrdRowSeatHeatgActlPwrCnsRiNgcc_read)
  ThrdRowSeatHeatgDesPwrCnsLeNgcc_ThrdRowSeatHeatgDesPwrCnsLeNgcc/1 -> ClimaEgyMgr_Runnable_sys/83(ThrdRowSeatHeatgDesPwrCnsLeNgcc_ThrdRowSeatHeatgDesPwrCnsLeNgcc_read)
  ThrdRowSeatHeatgDesPwrCnsRiNgcc_ThrdRowSeatHeatgDesPwrCnsRiNgcc/1 -> ClimaEgyMgr_Runnable_sys/84(ThrdRowSeatHeatgDesPwrCnsRiNgcc_ThrdRowSeatHeatgDesPwrCnsRiNgcc_read)
  ThrdRowSeatVentnActlPwrCnsLeNgcc_ThrdRowSeatVentnActlPwrCnsLeNgcc/1 -> ClimaEgyMgr_Runnable_sys/85(ThrdRowSeatVentnActlPwrCnsLeNgcc_ThrdRowSeatVentnActlPwrCnsLeNgcc_read)
  ThrdRowSeatVentnActlPwrCnsRiNgcc_ThrdRowSeatVentnActlPwrCnsRiNgcc/1 -> ClimaEgyMgr_Runnable_sys/86(ThrdRowSeatVentnActlPwrCnsRiNgcc_ThrdRowSeatVentnActlPwrCnsRiNgcc_read)
  ThrdRowSeatVentnDesPwrCnsLeNgcc_ThrdRowSeatVentnDesPwrCnsLeNgcc/1 -> ClimaEgyMgr_Runnable_sys/87(ThrdRowSeatVentnDesPwrCnsLeNgcc_ThrdRowSeatVentnDesPwrCnsLeNgcc_read)
  ThrdRowSeatVentnDesPwrCnsRiNgcc_ThrdRowSeatVentnDesPwrCnsRiNgcc/1 -> ClimaEgyMgr_Runnable_sys/88(ThrdRowSeatVentnDesPwrCnsRiNgcc_ThrdRowSeatVentnDesPwrCnsRiNgcc_read)
  TiAndDateIndcn_TiAndDateIndcn/1 -> ClimaEgyMgr_Runnable_sys/89(TiAndDateIndcn_TiAndDateIndcn_read)
  ClimaEgyMgr_Runnable_sys/1(ClimaBlwLimitedResult_ClimaBlwLimitedResult_write) -> ClimaBlwLimitedResult_ClimaBlwLimitedResult/1
  ClimaEgyMgr_Runnable_sys/2(DCDCPwrAllwdForHvacFanRe_DCDCPwrAllwdForHvacFanRe_write) -> DCDCPwrAllwdForHvacFanRe_DCDCPwrAllwdForHvacFanRe/1
  ClimaEgyMgr_Runnable_sys/3(FlowRednForEngStby_FlowRednForEngStby_write) -> FlowRednForEngStby_FlowRednForEngStby/1
  ClimaEgyMgr_Runnable_sys/4(FrntBlwWrkLmt_FrntBlwWrkLmt_write) -> FrntBlwWrkLmt_FrntBlwWrkLmt/1
  ClimaEgyMgr_Runnable_sys/5(MemSaveFlgForClimaEgyMgr_MemSaveFlgForClimaEgyMgr_write) -> MemSaveFlgForClimaEgyMgr_MemSaveFlgForClimaEgyMgr/1
  ClimaEgyMgr_Runnable_sys/6(MemWrForClimaEgyMgr_MemWrForClimaEgyMgr_write) -> MemWrForClimaEgyMgr_MemWrForClimaEgyMgr/1
  ClimaEgyMgr_Runnable_sys/7(OxygenSplyPwrAllwdNgcc_OxygenSplyPwrAllwdNgcc_write) -> OxygenSplyPwrAllwdNgcc_OxygenSplyPwrAllwdNgcc/1
  ClimaEgyMgr_Runnable_sys/8(ReBlwWrkLmt_ReBlwWrkLmt_write) -> ReBlwWrkLmt_ReBlwWrkLmt/1
  ClimaEgyMgr_Runnable_sys/9(ThrdRowLeSeatHeatgPwrAllwdNgcc_ThrdRowLeSeatHeatgPwrAllwdNgcc_write) -> ThrdRowLeSeatHeatgPwrAllwdNgcc_ThrdRowLeSeatHeatgPwrAllwdNgcc/1
  ClimaEgyMgr_Runnable_sys/10(ThrdRowLeSeatVentnPwrAllwdNgcc_ThrdRowLeSeatVentnPwrAllwdNgcc_write) -> ThrdRowLeSeatVentnPwrAllwdNgcc_ThrdRowLeSeatVentnPwrAllwdNgcc/1
  ClimaEgyMgr_Runnable_sys/11(ThrdRowRiSeatHeatgPwrAllwdNgcc_ThrdRowRiSeatHeatgPwrAllwdNgcc_write) -> ThrdRowRiSeatHeatgPwrAllwdNgcc_ThrdRowRiSeatHeatgPwrAllwdNgcc/1
  ClimaEgyMgr_Runnable_sys/12(ThrdRowRiSeatVentnPwrAllwdNgcc_ThrdRowRiSeatVentnPwrAllwdNgcc_write) -> ThrdRowRiSeatVentnPwrAllwdNgcc_ThrdRowRiSeatVentnPwrAllwdNgcc/1
  ClimaEgyMgr_Runnable_sys/13(ClimaPwrCns_Ngcc_ClimaPwrCns_write) -> ClimaPwrCns_Ngcc_ClimaPwrCns/1
  ClimaPwrCnsPred_ClimaPwrCnsPred_merge/1 -> ClimaPwrCnsPred_ClimaPwrCnsPred/1
  ClimaEgyMgr_Runnable_sys/15(ClimaPwrCritDes_Ngcc_ClimaPwrCritDes_write) -> ClimaPwrCritDes_Ngcc_ClimaPwrCritDes/1
  ClimaEgyMgr_Runnable_sys/16(ClimaPwrNormDes_Ngcc_ClimaPwrNormDes_write) -> ClimaPwrNormDes_Ngcc_ClimaPwrNormDes/1
  ClimaEgyMgr_Runnable_sys/17(CupHoldrActvAllwd_Ngcc_CupHoldrActvAllwd_write) -> CupHoldrActvAllwd_Ngcc_CupHoldrActvAllwd/1
  ClimaEgyMgr_Runnable_sys/18(DrvrSeatHeatgPwrAllwd_Ngcc_DrvrSeatHeatgPwrAllwd_write) -> DrvrSeatHeatgPwrAllwd_Ngcc_DrvrSeatHeatgPwrAllwd/1
  ClimaEgyMgr_Runnable_sys/19(DrvrSeatVentnPwrAllwd_Ngcc_DrvrSeatVentnPwrAllwd_write) -> DrvrSeatVentnPwrAllwd_Ngcc_DrvrSeatVentnPwrAllwd/1
  ClimaEgyMgr_Runnable_sys/20(FanPotiCtrldAllwd_FanPotiCtrldAllwd_write) -> FanPotiCtrldAllwd_FanPotiCtrldAllwd/1
  ClimaEgyMgr_Runnable_sys/21(FlowRednAtPwrAllwdLo_FlowRednAtPwrAllwdLo_write) -> FlowRednAtPwrAllwdLo_FlowRednAtPwrAllwdLo/1
  ClimaEgyMgr_Runnable_sys/22(FlowRednAtPwrLvlLo_FlowRednAtPwrLvlLo_write) -> FlowRednAtPwrLvlLo_FlowRednAtPwrLvlLo/1
  ClimaEgyMgr_Runnable_sys/23(FlowRednAtPwrLvlLo1_FlowRednAtPwrLvlLo1_write) -> FlowRednAtPwrLvlLo1_FlowRednAtPwrLvlLo1/1
  ClimaEgyMgr_Runnable_sys/24(PassSeatHeatgPwrAllwd_Ngcc_PassSeatHeatgPwrAllwd_write) -> PassSeatHeatgPwrAllwd_Ngcc_PassSeatHeatgPwrAllwd/1
  ClimaEgyMgr_Runnable_sys/25(PassSeatVentnPwrAllwd_Ngcc_PassSeatVentnPwrAllwd_write) -> PassSeatVentnPwrAllwd_Ngcc_PassSeatVentnPwrAllwd/1
  ClimaEgyMgr_Runnable_sys/26(PwrAllwdForAirFrag_PwrAllwdForAirFrag_write) -> PwrAllwdForAirFrag_PwrAllwdForAirFrag/1
  ClimaEgyMgr_Runnable_sys/27(PwrAllwdForElecAirVentn_Ngcc_PwrAllwdForElecAirVentn_write) -> PwrAllwdForElecAirVentn_Ngcc_PwrAllwdForElecAirVentn/1
  ClimaEgyMgr_Runnable_sys/28(PwrAllwdForHvacFanFrnt_PwrAllwdForHvacFanFrnt_write) -> PwrAllwdForHvacFanFrnt_PwrAllwdForHvacFanFrnt/1
  ClimaEgyMgr_Runnable_sys/29(PwrAllwdForHvacFanRe_PwrAllwdForHvacFanRe_write) -> PwrAllwdForHvacFanRe_PwrAllwdForHvacFanRe/1
  ClimaEgyMgr_Runnable_sys/30(PwrAllwdForHvacHeatrElecFrnt_PwrAllwdForHvacHeatrElecFrnt_write) -> PwrAllwdForHvacHeatrElecFrnt_PwrAllwdForHvacHeatrElecFrnt/1
  ClimaEgyMgr_Runnable_sys/31(PwrAllwdForHvacHeatrElecRe_PwrAllwdForHvacHeatrElecRe_write) -> PwrAllwdForHvacHeatrElecRe_PwrAllwdForHvacHeatrElecRe/1
  ClimaEgyMgr_Runnable_sys/32(PwrAllwdForSeatHeatg_PwrAllwdForSeatHeatg_write) -> PwrAllwdForSeatHeatg_PwrAllwdForSeatHeatg/1
  ClimaEgyMgr_Runnable_sys/33(PwrAllwdForSeatVent_PwrAllwdForSeatVent_write) -> PwrAllwdForSeatVent_PwrAllwdForSeatVent/1
  ClimaEgyMgr_Runnable_sys/34(PwrEstimdAtStbForClima_Ngcc_PwrEstimdAtStbForClima_write) -> PwrEstimdAtStbForClima_Ngcc_PwrEstimdAtStbForClima/1
  ClimaEgyMgr_Runnable_sys/35(SecRowLeSeatHeatgPwrAllwd_Ngcc_SecRowLeSeatHeatgPwrAllwd_write) -> SecRowLeSeatHeatgPwrAllwd_Ngcc_SecRowLeSeatHeatgPwrAllwd/1
  ClimaEgyMgr_Runnable_sys/36(SecRowLeSeatVentnPwrAllwd_Ngcc_SecRowLeSeatVentnPwrAllwd_write) -> SecRowLeSeatVentnPwrAllwd_Ngcc_SecRowLeSeatVentnPwrAllwd/1
  ClimaEgyMgr_Runnable_sys/37(SecRowRiSeatHeatgPwrAllwd_Ngcc_SecRowRiSeatHeatgPwrAllwd_write) -> SecRowRiSeatHeatgPwrAllwd_Ngcc_SecRowRiSeatHeatgPwrAllwd/1
  ClimaEgyMgr_Runnable_sys/38(SecRowRiSeatVentnPwrAllwd_Ngcc_SecRowRiSeatVentnPwrAllwd_write) -> SecRowRiSeatVentnPwrAllwd_Ngcc_SecRowRiSeatVentnPwrAllwd/1
  ClimaEgyMgr_Runnable_sys/39(SteerWhlHeatgAllwd_SteerWhlHeatgAllwd_write) -> SteerWhlHeatgAllwd_SteerWhlHeatgAllwd/1
  ClimaEgyMgr_Runnable_sys/40(SteerWhlHeatgPwrAllwd_SteerWhlHeatgPwrAllwd_write) -> SteerWhlHeatgPwrAllwd_SteerWhlHeatgPwrAllwd/1
  ClimaEgyMgr_Runnable_sys/14(ClimaPwrCnsPred_ClimaPwrCnsPred_write) -> [ClimaPwrCnsPred_ClimaPwrCnsPred_merge_Tag]
  [ClimaPwrCnsPred_ClimaPwrCnsPred_merge_Tag] -> ClimaPwrCnsPred_ClimaPwrCnsPred_merge/2
  ClimaEgyMgr_Init/1(ClimaPwrCnsPred_ClimaPwrCnsPred_write) -> [ClimaPwrCnsPred_ClimaPwrCnsPred_merge_Tag1]
  [ClimaPwrCnsPred_ClimaPwrCnsPred_merge_Tag1] -> ClimaPwrCnsPred_ClimaPwrCnsPred_merge/1
  ClimaEgyMgr_Runnable/1 -> ClimaEgyMgr_Runnable_sys/Trigger

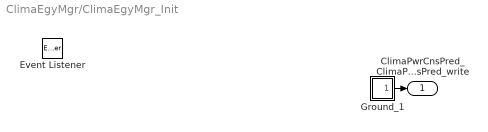
== system ClimaEgyMgr/ClimaEgyMgr_Init ==
  Event Listener : EventListener  EventName=reset
  Ground_1 : Subsystem
  ClimaPwrCnsPred_ClimaPwrCnsPred_write : Outport
  wires:
  Ground_1/1(Out1) -> ClimaPwrCnsPred_ClimaPwrCnsPred_write/1
